simulink model "Simulink_Program"  (R2023b)
settings: Solver=FixedStepAuto  StartTime=0.0  StopTime=20  FixedStep=0.001  RelTol=1e-3
PreLoadFcn:
  | % NXP_MBDT_S32K3 callback: DO NOT REMOVE
  | mbd_s32k3.common.nxp.model_callbacks.preLoadFcn();
PostLoadFcn:
  | % NXP_MBDT_S32K3 callback: DO NOT REMOVE
  | mbd_s32k3.common.nxp.model_callbacks.postLoadFcn();
InitFcn:
  | % NXP_MBDT_S32K3 callback: DO NOT REMOVE
  | mbd_s32k3.common.nxp.model_callbacks.initFcn();
undefined symbols (not defined in the file or in its folder): battery_builder_rtmsupport, DiscIntNeverNeededParam, gcbh
libraries: nesl_utility x116, pack_105s34p_structured_with_balancing_lib x105, fl_lib x16, ee_lib x7, simulink x5, BatteryBalancing x1
(part 1 of 8)
settings Model: DataDictionary=Simulink_Program.sldd  Creator=romul  LastModifiedBy=mcmas
settings OptimizationCC: EnhancedBackFolding=on  PassReuseOutputArgsAs=Individual arguments  ZeroExternalMemoryAtStartup=off  ZeroInternalMemoryAtStartup=off  MaxStackSize=64  OptimizeBlockOrder=speed  DifferentSizesBufferReuse=on  OptimizationCustomize=off  EfficientTunableParamExpr=on
settings DebuggingCC: ParameterTunabilityLossMsg=error
settings HardwareCC: ProdBitPerPointer=32  ProdBitPerSizeT=32  ProdBitPerPtrDiffT=32  ProdLargestAtomicInteger=Long  ProdLargestAtomicFloat=Double  ProdWordSize=32  ProdHWDeviceType=ARM Compatible->ARM Cortex
settings SFSimCC: MATLABDynamicMemAlloc=off
settings RTWCC: Description=Embedded Coder  SystemTargetFile=ert.tlc  HardwareBoard=NXP S32K3xx  TemplateMakefile=ert_default_tmf  Toolchain=S32DS GCC
settings CodeAppCC: ForceParamTrailComments=on  IgnoreCustomStorageClasses=off  OperatorAnnotations=on  SimulinkDataObjDesc=on  SFDataObjDesc=on  InsertBlockDesc=on
settings ERTTargetCC: ExtModeTransport=1  ExtModeMexFile=ext_serial_win32_comm  ERTSrcFileBannerTemplate=mbd_s32k3_ert_code_template.cgt  ERTHdrFileBannerTemplate=mbd_s32k3_ert_code_template.cgt  ERTDataSrcFileTemplate=mbd_s32k3_ert_code_template.cgt  ERTDataHdrFileTemplate=mbd_s32k3_ert_code_template.cgt

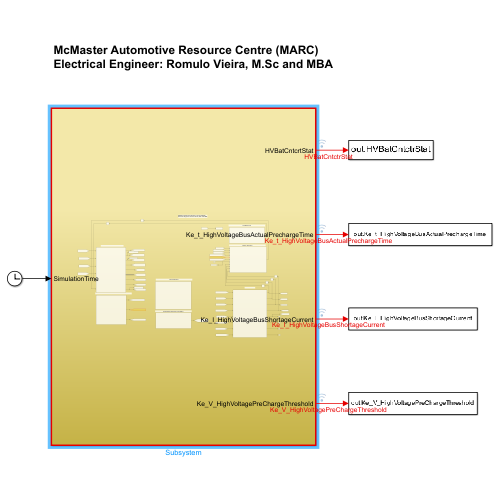
[thumbnail: Simulink's stored preview of the top-level diagram]
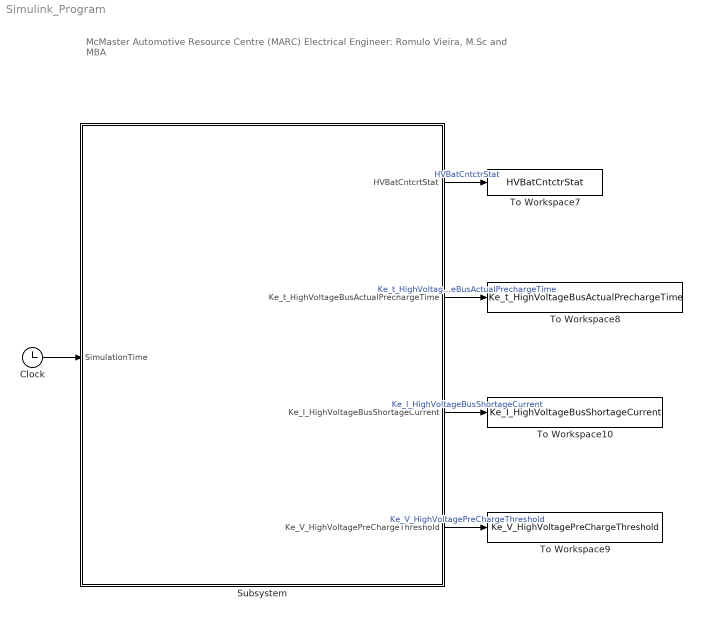
== system Simulink_Program ==
  note: McMaster Automotive Resource Centre (MARC)
      | Electrical Engineer: Romulo Vieira, M.Sc and MBA
  Clock : Clock
  Subsystem : Subsystem  TreatAsAtomicUnit=on
  To Workspace10 : ToWorkspace  VariableName=Ke_I_HighVoltageBusShortageCurrent  SampleTime=-1
  To Workspace7 : ToWorkspace  VariableName=HVBatCntctrStat  SampleTime=-1
  To Workspace8 : ToWorkspace  VariableName=Ke_t_HighVoltageBusActualPrechargeTime  SampleTime=-1
  To Workspace9 : ToWorkspace  VariableName=Ke_V_HighVoltagePreChargeThreshold  SampleTime=-1
  wires:
  Subsystem/4(Ke_V_HighVoltagePreChargeThreshold) -> To Workspace9/1  "Ke_V_HighVoltagePreChargeThreshold"
  Subsystem/2(Ke_t_HighVoltageBusActualPrechargeTime) -> To Workspace8/1  "Ke_t_HighVoltageBusActualPrechargeTime"
  Subsystem/1(HVBatCntcrtStat) -> To Workspace7/1  "HVBatCntctrStat"
  Subsystem/3(Ke_I_HighVoltageBusShortageCurrent) -> To Workspace10/1  "Ke_I_HighVoltageBusShortageCurrent"
  Clock/1 -> Subsystem/1(SimulationTime)

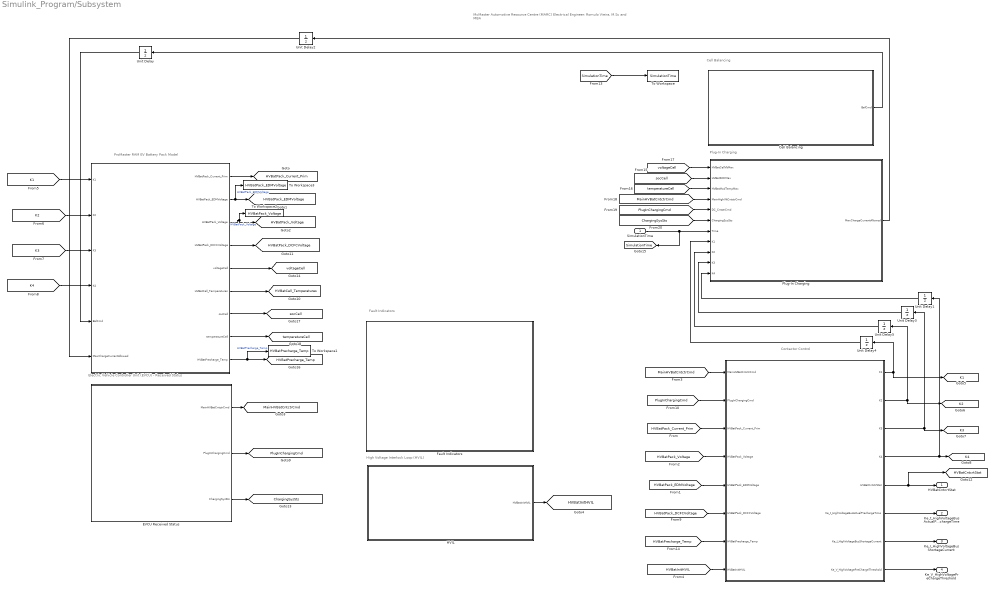
== system Simulink_Program/Subsystem ==
  note: McMaster Automotive Resource Centre (MARC)
      | Electrical Engineer: Romulo Vieira, M.Sc and MBA
  note: ProMaster RAM EV Battery Pack Model
  note: Contactor Control
  note: Plug-In Charging
  note: Cell Balancing
  note: High Voltage Interlock Loop (HVIL)
  note: Fault Indicators
  note: Electric Vehicle Controller Unit (EVCU) - Received Status
  SimulationTime : Inport
  " " : Subsystem  TreatAsAtomicUnit=on  SystemSampleTime=0.001
  "  " : Subsystem  TreatAsAtomicUnit=on  SystemSampleTime=0.001
  Cell Balancing : Subsystem
  EVCU Received Status : Subsystem
  Fault Indicators : Subsystem
  HVIL : Subsystem
  Plug-In Charging : Subsystem  TreatAsAtomicUnit=on  SystemSampleTime=0.001
  To Workspace : ToWorkspace  VariableName=SimulationTime  SampleTime=-1
  To Workspace1 : ToWorkspace  VariableName=HVBatPrecharge_Temp  SampleTime=-1
  To Workspace2 : ToWorkspace  VariableName=HVBatPack_Voltage  SampleTime=-1
  To Workspace3 : ToWorkspace  VariableName=HVBatPack_EDMVoltage  SampleTime=-1
  Unit Delay{,1..5} (6 alike) : UnitDelay  SampleTime=-1  HasFrameUpgradeWarning=on
  HVBatCntcrtStat : Outport
  Ke_t_HighVoltageBusActualPrechargeTime : Outport  Port=2
  Ke_I_HighVoltageBusShortageCurrent : Outport  Port=3
  Ke_V_HighVoltagePreChargeThreshold : Outport  Port=4
  tag scope: ChargingSysSts=global, HVBatCell_Temperatures=global, HVBatCntcrtStat=global, HVBatInitHVIL=global, HVBatPack_Current_Prim=global, HVBatPack_DCFCVoltage=global, HVBatPack_EDMVoltage=global, HVBatPack_Voltage=global, HVBatPrecharge_Temp=global, K1=global, K2=global, K3=global, K4=global, MainHVBatCntctrCmd=global, PlugInChargingCmd=global, SimulationTime=global, socCell=global, temperatureCell=global, voltageCell=global
  wires:
  "  "/5(HVBatCntctrStat) -> [HVBatCntcrtStat], HVBatCntcrtStat/1
  "  "/6(Ke_t_HighVoltageBusActualPrechargeTime) -> Ke_t_HighVoltageBusActualPrechargeTime/1
  "  "/7(Ke_I_HighVoltageBusShortageCurrent) -> Ke_I_HighVoltageBusShortageCurrent/1
  "  "/8(Ke_V_HighVoltagePreChargeThreshold) -> Ke_V_HighVoltagePreChargeThreshold/1
  " "/1(HVBatPack_Current_Prim) -> [HVBatPack_Current_Prim]
  " "/2(HVBatPack_EDMVoltage) -> To Workspace3/1, [HVBatPack_EDMVoltage]  "HVBatPack_EDMVoltage"
  " "/3(HVBatPack_Voltage) -> To Workspace2/1, [HVBatPack_Voltage]  "HVBatPack_Voltage"
  [HVBatPack_Current_Prim] -> "  "/3(HVBatPack_Current_Prim)
  [HVBatPack_EDMVoltage] -> "  "/5(HVBatPack_EDMVoltage)
  [HVBatPack_Voltage] -> "  "/4(HVBatPack_Voltage)
  EVCU Received Status/1(" MainHVBatCntctrCmd      ") -> [MainHVBatCntctrCmd]
  [MainHVBatCntctrCmd] -> "  "/1(MainHVBatCntctrCmd)
  HVIL/1("HVBatInitHVIL   ") -> [HVBatInitHVIL]
  [HVBatInitHVIL] -> "  "/8(HVBatInitHVIL)
  "  "/3(K3) -> [K3], Unit Delay3/1
  "  "/4(K4) -> Unit Delay1/1, [K4]
  [K2] -> " "/2(K2)
  [K3] -> " "/3(K3)
  [K4] -> " "/4(K4)
  " "/4(HVBatPack_DCFCVoltage) -> [HVBatPack_DCFCVoltage]
  [HVBatPack_DCFCVoltage] -> "  "/6(HVBatPack_DCFCVoltage)
  [PlugInChargingCmd] -> "  "/2(PlugInChargingCmd)
  EVCU Received Status/2(" PlugInChargingCmd      ") -> [PlugInChargingCmd]
  " "/5(voltageCell) -> [voltageCell]
  " "/6(HVBatCell_Temperatures) -> [HVBatCell_Temperatures]
  " "/9(HVBatPrecharge_Temp) -> To Workspace1/1, [HVBatPrecharge_Temp]  "HVBatPrecharge_Temp"
  [K1] -> " "/1(K1)
  [SimulationTime] -> To Workspace/1
  SimulationTime/1 -> [SimulationTime], Plug-In Charging/7(Time)
  Cell Balancing/1(BalCmd) -> Unit Delay/1
  Unit Delay/1 -> " "/5(BalCmd)
  Unit Delay1/1 -> Plug-In Charging/11(K4)
  EVCU Received Status/3(" ChargingSysSts") -> [ChargingSysSts]
  [HVBatPrecharge_Temp] -> "  "/7(HVBatPrecharge_Temp)
  Unit Delay3/1 -> Plug-In Charging/10(K3)
  Unit Delay5/1 -> Plug-In Charging/9(K2)
  "  "/2(K2) -> [K2], Unit Delay5/1
  Unit Delay4/1 -> Plug-In Charging/8(K1)
  "  "/1(K1) -> [K1], Unit Delay4/1
  " "/7(socCell) -> [socCell]
  " "/8(temperatureCell) -> [temperatureCell]
  Plug-In Charging/1(MaxChargeCurrentAllowed) -> Unit Delay2/1
  Unit Delay2/1 -> " "/6(MaxChargeCurrentAllowed)
  [voltageCell] -> Plug-In Charging/1(HVBatCellVltMax)
  [socCell] -> Plug-In Charging/2(HVBatSOCMax)
  [temperatureCell] -> Plug-In Charging/3(HVBatModTempMax)
  [MainHVBatCntctrCmd] -> Plug-In Charging/4(MainHighVltCntctrCmd)
  [PlugInChargingCmd] -> Plug-In Charging/5(DC_CntctrCmd)
  [ChargingSysSts] -> Plug-In Charging/6(ChargingSysSts)

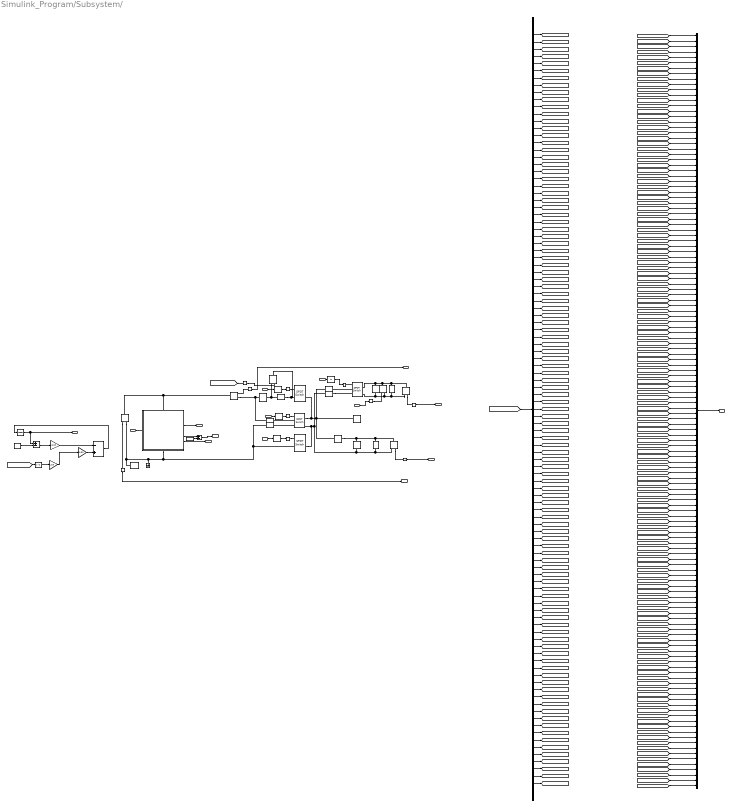
== system Simulink_Program/Subsystem/  ==
  note: McMaster Automotive Resource Centre (MARC)
      | Electrical Engineer: Romulo Vieira, M.Sc and MBA
  note: Bus Creator for cell temperatures
  note: Demux to separate 105 temperatures
  note: Measuring precharge resistor temperature
      | 1. Measure Voltage Across the Precharge Resistor using a voltage sensor.
      | 2. Calculate Power Dissipated in the Resistor. Use P(t) = V(t)^2 / R where precharge resistance, R = 25 ohm
      | 3. Model the Thermal Behaviour. Use dT/dt = P(t)/C - (T(t) - T_ambient)/(R_th*C) where thermal capacitance, C = 50 J/deg C, thermal resistance, R_th = 2 deg C/W and ambient temperature, T_ambient = 25 deg C
      | 4. Use an integrator to output the final temperature value.
  note: Input
      | precharge_resistor_voltage
      |
      | Output
      | precharge_resistor_temperature
  K1 : Inport
  K2 : Inport  Port=2
  K3 : Inport  Port=3
  K4 : Inport  Port=4
  BalCmd : Inport  Port=5
  MaxChargeCurrentAllowed : Inport  Port=6
  "   " : nesl_utility/PS-Simulink Converter  PhysicalDomain=network_engine_domain  SubClassName=ps_output  LeftPortType=input  RightPortType=output  PseudoPeriodic=off  Frequencies=[]  VectorFormat=inherit  Unit=inherit  AffineConversion=off
  " " : nesl_utility/PS-Simulink Converter  PhysicalDomain=network_engine_domain  SubClassName=ps_output  LeftPortType=input  RightPortType=output  PseudoPeriodic=off  Frequencies=[]  VectorFormat=inherit  Unit=inherit  AffineConversion=off
  " 1" : nesl_utility/PS-Simulink Converter  PhysicalDomain=network_engine_domain  SubClassName=ps_output  LeftPortType=input  RightPortType=output  PseudoPeriodic=off  Frequencies=[]  VectorFormat=inherit  Unit=inherit  AffineConversion=off
  1/(R_th*C) : Gain  Gain=1/100
  1/C : Gain  Gain=1/50
  1/R : Gain  Gain=1/25
  BusCreator_Temp : BusCreator  Inputs=140
  Capacitor : fl_lib/Electrical/Electrical Elements/Capacitor  c=750e-6 F  r=1e-6 Ohm  g=0 1/Ohm  i_specify=off  i_priority=None  i=0 A  i_nominal_specify=off  i_nominal_unit=A  i_nominal_value=1  v_specify=off  v_priority=None  v=0 V  v_nominal_specify=off  v_nominal_unit=V  v_nominal_value=1  vc_specify=off  vc_priority=High  vc=0 V  vc_nominal_specify=off  vc_nominal_unit=V  vc_nominal_value=1
  Capacitor Bank : fl_lib/Electrical/Electrical Elements/Capacitor  c=750e-6 F  r=1e-6 Ohm  g=0 1/Ohm  i_specify=off  i_priority=None  i=0 A  i_nominal_specify=off  i_nominal_unit=A  i_nominal_value=1  v_specify=off  v_priority=None  v=0 V  v_nominal_specify=off  v_nominal_unit=V  v_nominal_value=1  vc_specify=off  vc_priority=High  vc=441 V  vc_nominal_specify=off  vc_nominal_unit=V  vc_nominal_value=1
  Constant : Constant  Value=273.15
  Controlled Current Source : fl_lib/Electrical/Electrical Sources/Controlled Current Source
  Current Sensor : fl_lib/Electrical/Electrical Sensors/Current Sensor
  Delay : Delay  DelayLength=1
  Delay4 : Delay  DelayLength=1
  Delay5 : Delay  DelayLength=1
  Demux1 : Demux  Outputs=105
  Discrete-Time Integrator : DiscreteIntegrator  InitialCondition=25  SampleTime=0.001  ICPrevOutput=DiscIntNeverNeededParam  ICPrevScaledInput=DiscIntNeverNeededParam
  F2 - PDC Fuse : ee_lib/Switches & Breakers/Fuse  prm=1  I_rated=125 A  melt_margin=1  T_fuse=0 s  I2t=1 A^2*s  I_vec=[1.9, 2, 2.5, 30] A  t_vec=[10000, 1, .3, .001] s  R_closed=0.01 Ohm  G_open=1e-8 1/Ohm  exposemeasurement=ee.enum.exposemeasurement.hidden
      settings: InternalSimscapePortConfiguration="{\"Bottom\":[],\"Left\":[{\"id\":\"p\",\"label\":\"\",\"type\":\"foundation.electrical.electrical\"}],\"Right\":[{\"id\":\"n\",\"label\":\"\",\"type\":\"foundation.electrical.electrical\"}],\"Top\":[]}"
  F3 - EDM Fuse : ee_lib/Switches & Breakers/Fuse  prm=1  I_rated=600 A  melt_margin=1  T_fuse=0 s  I2t=1 A^2*s  I_vec=[1.9, 2, 2.5, 30] A  t_vec=[10000, 1, .3, .001] s  R_closed=0.01 Ohm  G_open=1e-8 1/Ohm  exposemeasurement=ee.enum.exposemeasurement.hidden
      settings: InternalSimscapePortConfiguration="{\"Bottom\":[],\"Left\":[{\"id\":\"p\",\"label\":\"\",\"type\":\"foundation.electrical.electrical\"}],\"Right\":[{\"id\":\"n\",\"label\":\"\",\"type\":\"foundation.electrical.electrical\"}],\"Top\":[]}"
  Ground : fl_lib/Electrical/Electrical Elements/Electrical Reference
  K1 - Main Contactor : ee_lib/Switches & Breakers/DPDT Switch  R_closed=0.01 Ohm  G_open=1e-6 S  Threshold=0.5 V  Width=0 V  model_dynamics=0  t_on=1e-3 s  t_off=1e-3 s  initial_vT=0 V
      settings: InternalSimscapePortConfiguration="{\"Bottom\":[],\"Left\":[{\"id\":\"vT\",\"label\":\"vT\",\"type\":\"input\"},{\"id\":\"p1\",\"label\":\"\",\"type\":\"foundation.electrical.electrical\"},{\"id\":\"p2\",\"label\":\"\",\"type\":\"foundation.electrical.electrical\"}],\"Right\":[{\"id\":\"n11\",\"label\":\"\",\"type\":\"foundation.electrical.electrical\"},{\"id\":\"n12\",\"label\":\"\",\"type\":\"foundation.electrical.electrical\"},{\"id\":\"n21\",\"label\":\"\",\"type\":\"foundation.electrical.electrical\"},{\"id\":\"n22\",\"label\":\"\",\"type\":\"foundation.electrical.electrical\"}],\"Top\":[]}"
  K2 - Precharge Relay (-) : ee_lib/Switches & Breakers/SPDT Switch  R_closed=0.01 Ohm  G_open=1e-6 S  Threshold=0.5 V  Width=0 V  model_dynamics=0  t_on=1e-3 s  t_off=1e-3 s  initial_vT=0 V
  K3 - Precharge Relay (+) : ee_lib/Switches & Breakers/SPDT Switch  R_closed=0.01 Ohm  G_open=1e-6 S  Threshold=0.5 V  Width=0 V  model_dynamics=0  t_on=1e-3 s  t_off=1e-3 s  initial_vT=0 V
  K4 - DCFC Contactor : ee_lib/Switches & Breakers/DPDT Switch  R_closed=0.01 Ohm  G_open=1e-6 S  Threshold=0.5 V  Width=0 V  model_dynamics=0  t_on=1e-3 s  t_off=1e-3 s  initial_vT=0 V
      settings: InternalSimscapePortConfiguration="{\"Bottom\":[],\"Left\":[{\"id\":\"vT\",\"label\":\"vT\",\"type\":\"input\"},{\"id\":\"p1\",\"label\":\"\",\"type\":\"foundation.electrical.electrical\"},{\"id\":\"p2\",\"label\":\"\",\"type\":\"foundation.electrical.electrical\"}],\"Right\":[{\"id\":\"n11\",\"label\":\"\",\"type\":\"foundation.electrical.electrical\"},{\"id\":\"n12\",\"label\":\"\",\"type\":\"foundation.electrical.electrical\"},{\"id\":\"n21\",\"label\":\"\",\"type\":\"foundation.electrical.electrical\"},{\"id\":\"n22\",\"label\":\"\",\"type\":\"foundation.electrical.electrical\"}],\"Top\":[]}"
  K4 Resistor (Pole 1) : fl_lib/Electrical/Electrical Elements/Resistor  R=0.0003 Ohm  i_specify=off  i_priority=None  i=0 A  i_nominal_specify=off  i_nominal_unit=A  i_nominal_value=1  v_specify=off  v_priority=None  v=0 V  v_nominal_specify=off  v_nominal_unit=V  v_nominal_value=1
  K4 Resistor (Pole 2) : fl_lib/Electrical/Electrical Elements/Resistor  R=0.0003 Ohm  i_specify=off  i_priority=None  i=0 A  i_nominal_specify=off  i_nominal_unit=A  i_nominal_value=1  v_specify=off  v_priority=None  v=0 V  v_nominal_specify=off  v_nominal_unit=V  v_nominal_value=1
  PS-Simulink 1 : nesl_utility/PS-Simulink Converter  PhysicalDomain=network_engine_domain  SubClassName=ps_output  LeftPortType=input  RightPortType=output  PseudoPeriodic=off  Frequencies=[]  VectorFormat=inherit  Unit=inherit  AffineConversion=off
  PS-Simulink Converter : nesl_utility/PS-Simulink Converter  PhysicalDomain=network_engine_domain  SubClassName=ps_output  LeftPortType=input  RightPortType=output  PseudoPeriodic=off  Frequencies=[]  VectorFormat=inherit  Unit=inherit  AffineConversion=off
  Pack1 : Subsystem  LoadFcn=battery_builder_rtmsupport('loadfcn',gcbh);
      settings: CopyFcn=battery_builder_rtmsupport('copyfcn',gcbh);  PreCopyFcn=battery_builder_rtmsupport('precopyfcn',gcbh);  PreDeleteFcn=battery_builder_rtmsupport('predeletefcn',gcbh);  PortSchema="{\"entries\":[{\"content\":{\"sides\":[{\"content\":{\"connectorIds\":[\"LConn1\"]},\"type\":\"ConnectorPlacement.EquallySpacedRectSide\",\"uuid\":\"5e007e92-2246-4b9f-a838-21fe4b771105\"},{\"content\":{\"connectorIds\":[\"RConn1\"],\"side\":\"RIGHT\"},\"type\":\"ConnectorPlacement.EquallySpacedRectSide\",\"uuid\":\"c53269da-b12c-4944-8bcf-f548990142fd\"},{\"content\":{\"connectorIds\":[\"In1\"],\"side\":\"TOP\"},\"type\":\"ConnectorPlacement.EquallySpacedRectSide\",\"uuid\":\"229c8070-2bae-4d06-bc1c-b3e24a21fda1\"},{\"content\":{\"connectorIds\":[\"Out1\",\"Out2\",\"Out3\",\"Out4\",\"Out5\",\"Out6\",\"Out7\"],\"side\":\"BOTTOM\"},\"type\":\"ConnectorPlacement.EquallySpacedRectSide\",\"uuid\":\"01f05c69-bd27-4a3f-8a69-5e0eef156d08\"}]},\"type\":\"ConnectorPlacement.EquallySpacedConnector\",\"uuid\":\"d4cf6711-9d91-4f90-9da8-75b40740f7c2\"}],\"packageUris\":[\"http://schema.mathworks.com/mf0/Connector/R2020b\"],\"version\":\"1.0\"}"
      mask Display: image('$imagefile')
  Precharge Fuse : ee_lib/Switches & Breakers/Fuse  prm=1  I_rated=600 A  melt_margin=1  T_fuse=0 s  I2t=1 A^2*s  I_vec=[1.9, 2, 2.5, 30] A  t_vec=[10000, 1, .3, .001] s  R_closed=0.01 Ohm  G_open=1e-8 1/Ohm  exposemeasurement=ee.enum.exposemeasurement.hidden
      settings: InternalSimscapePortConfiguration="{\"Bottom\":[],\"Left\":[{\"id\":\"p\",\"label\":\"\",\"type\":\"foundation.electrical.electrical\"}],\"Right\":[{\"id\":\"n\",\"label\":\"\",\"type\":\"foundation.electrical.electrical\"}],\"Top\":[]}"
  Precharge Resistor : fl_lib/Electrical/Electrical Elements/Resistor  R=25 Ohm  i_specify=off  i_priority=None  i=0 A  i_nominal_specify=off  i_nominal_unit=A  i_nominal_value=1  v_specify=off  v_priority=None  v=0 V  v_nominal_specify=off  v_nominal_unit=V  v_nominal_value=1
  Resistor : fl_lib/Electrical/Electrical Elements/Resistor  R=1 Ohm  i_specify=off  i_priority=None  i=0 A  i_nominal_specify=off  i_nominal_unit=A  i_nominal_value=1  v_specify=off  v_priority=None  v=0 V  v_nominal_specify=off  v_nominal_unit=V  v_nominal_value=1
  Resistor1 : fl_lib/Electrical/Electrical Elements/Resistor  R=1 Ohm  i_specify=off  i_priority=None  i=0 A  i_nominal_specify=off  i_nominal_unit=A  i_nominal_value=1  v_specify=off  v_priority=None  v=0 V  v_nominal_specify=off  v_nominal_unit=V  v_nominal_value=1
  Resistor2 : fl_lib/Electrical/Electrical Elements/Resistor  R=40 kOhm  i_specify=off  i_priority=None  i=0 A  i_nominal_specify=off  i_nominal_unit=A  i_nominal_value=1  v_specify=off  v_priority=None  v=0 V  v_nominal_specify=off  v_nominal_unit=V  v_nominal_value=1
  Resistor3 : fl_lib/Electrical/Electrical Elements/Resistor  R=40 kOhm  i_specify=off  i_priority=None  i=0 A  i_nominal_specify=off  i_nominal_unit=A  i_nominal_value=1  v_specify=off  v_priority=None  v=0 V  v_nominal_specify=off  v_nominal_unit=V  v_nominal_value=1
  Simulink-PS Converter{,1..4} (5 alike) : nesl_utility/Simulink-PS Converter  PhysicalDomain=network_engine_domain  SubClassName=ps_input  LeftPortType=input  RightPortType=output  PseudoPeriodic=off  Frequencies=[]  NoiseDistribution=none  NoiseParameters=[]  Unit=1  AffineConversion=off  FilteringAndDerivatives=provide  SimscapeFilterOrder=1  InputFilterTimeConstant=0.001  UdotUserProvided=0
  Solver Configuration : nesl_utility/Solver Configuration  PhysicalDomain=network_engine_domain  SubClassName=solver  LeftPortType=input  RightPortType=generic  Tones=[]  Harmonics=[]  SimulateNoise=off  Profile=off  UseLocalSampling=off  EnableSwitchedLinearOptims=on  FrequencyDomain=off  UseCCode=off  RelTol=0.001  AbsTol=0.001  MinStep=1e-09  ToWorkspace=off  RevertToSquareIcSolve=off  NumHomotopyIterations=100  PhasorMode=off  PhasorModeNumHarmonics=1  ConsistencySolver=NEWTON_XTOL_AFTER_FTOL  IndexReductionMethod=DerivativeReplacement  DoDC=off  ResidualTolerance=1e-09  ConsistencyTolSource=GLOBAL  ConsistencyAbsTol=0.001  ConsistencyRelTol=0.001  ConsistencyTolFactor=0.001  UseLocalSolver=on  LocalSolverChoice=NE_BACKWARD_EULER_ADVANCER  ResolveIndetEquations=on  FunctionEvalNumThread=1  LocalSolverSampleTime=0.001  DoFixedCost=on  MaxNonlinIter=3  MaxModeIter=2  ComputeImpulses=off  ImpulseIterations=2  LinearAlgebra=auto  LinearAlgebraNumThread=0  EquationFormulation=NE_TIME_EF  DelaysMemoryBudget=1024  AutomaticFiltering=off  FilteringTimeConstant=0.001  PartitionStorageMethod=AS_NEEDED  PartitionMemoryBudget=1024  PartitionMethod=ROBUST  MultibodyUseLocalSolver=off  MultibodyLocalSolverChoice=ODE1  MultibodyLocalSolverSampleTime=0.001
  Subtract : Sum  Inputs=-+
  Subtract1 : Sum  Inputs=+-
  T(t) - T_ambient : Sum  Inputs=+-
  T_ambient : Constant  Value=25
  Unit Delay : UnitDelay  SampleTime=-1  HasFrameUpgradeWarning=on
  V^2 : Math  Operator=square
  Voltage Sensor{,1..3} (4 alike) : fl_lib/Electrical/Electrical Sensors/Voltage Sensor
  HVBatPack_Current_Prim : Outport
  HVBatPack_EDMVoltage : Outport  Port=2
  HVBatPack_Voltage : Outport  Port=3
  HVBatPack_DCFCVoltage : Outport  Port=4
  voltageCell : Outport  Port=5
  HVBatCell_Temperatures : Outport  Port=6
  socCell : Outport  Port=7
  temperatureCell : Outport  Port=8
  HVBatPrecharge_Temp : Outport  Port=9
  tag scope: HVBatCell_Temperature001=global, HVBatCell_Temperature002=global, HVBatCell_Temperature003=global, HVBatCell_Temperature004=global, HVBatCell_Temperature005=global, HVBatCell_Temperature006=global, HVBatCell_Temperature007=global, HVBatCell_Temperature008=global, HVBatCell_Temperature009=global, HVBatCell_Temperature010=global, HVBatCell_Temperature011=global, HVBatCell_Temperature012=global, HVBatCell_Temperature013=global, HVBatCell_Temperature014=global, HVBatCell_Temperature015=global, HVBatCell_Temperature016=global, HVBatCell_Temperature017=global, HVBatCell_Temperature018=global, HVBatCell_Temperature019=global, HVBatCell_Temperature020=global, HVBatCell_Temperature021=global, HVBatCell_Temperature022=global, HVBatCell_Temperature023=global, HVBatCell_Temperature024=global, HVBatCell_Temperature025=global, HVBatCell_Temperature026=global, HVBatCell_Temperature027=global, HVBatCell_Temperature028=global, HVBatCell_Temperature029=global, HVBatCell_Temperature030=global, HVBatCell_Temperature031=global, HVBatCell_Temperature032=global, HVBatCell_Temperature033=global, HVBatCell_Temperature034=global, HVBatCell_Temperature035=global, HVBatCell_Temperature036=global, HVBatCell_Temperature037=global, HVBatCell_Temperature038=global, HVBatCell_Temperature039=global, HVBatCell_Temperature040=global, HVBatCell_Temperature041=global, HVBatCell_Temperature042=global, HVBatCell_Temperature043=global, HVBatCell_Temperature044=global, HVBatCell_Temperature045=global, HVBatCell_Temperature046=global, HVBatCell_Temperature047=global, HVBatCell_Temperature048=global, HVBatCell_Temperature049=global, HVBatCell_Temperature050=global, HVBatCell_Temperature051=global, HVBatCell_Temperature052=global, HVBatCell_Temperature053=global, HVBatCell_Temperature054=global, HVBatCell_Temperature055=global, HVBatCell_Temperature056=global, HVBatCell_Temperature057=global, HVBatCell_Temperature058=global, HVBatCell_Temperature059=global, HVBatCell_Temperature060=global, HVBatCell_Temperature061=global, HVBatCell_Temperature062=global, HVBatCell_Temperature063=global, HVBatCell_Temperature064=global, HVBatCell_Temperature065=global, HVBatCell_Temperature066=global, HVBatCell_Temperature067=global, HVBatCell_Temperature068=global, HVBatCell_Temperature069=global, HVBatCell_Temperature070=global, HVBatCell_Temperature071=global, HVBatCell_Temperature072=global, HVBatCell_Temperature073=global, HVBatCell_Temperature074=global, HVBatCell_Temperature075=global, HVBatCell_Temperature076=global, HVBatCell_Temperature077=global, HVBatCell_Temperature078=global, HVBatCell_Temperature079=global, HVBatCell_Temperature080=global, HVBatCell_Temperature081=global, HVBatCell_Temperature082=global, HVBatCell_Temperature083=global, HVBatCell_Temperature084=global, HVBatCell_Temperature085=global, HVBatCell_Temperature086=global, HVBatCell_Temperature087=global, HVBatCell_Temperature088=global, HVBatCell_Temperature089=global, HVBatCell_Temperature090=global, HVBatCell_Temperature091=global, HVBatCell_Temperature092=global, HVBatCell_Temperature093=global, HVBatCell_Temperature094=global, HVBatCell_Temperature095=global, HVBatCell_Temperature096=global, HVBatCell_Temperature097=global, HVBatCell_Temperature098=global, HVBatCell_Temperature099=global, HVBatCell_Temperature100=global, HVBatCell_Temperature101=global, HVBatCell_Temperature102=global, HVBatCell_Temperature103=global, HVBatCell_Temperature104=global, HVBatCell_Temperature105=global, HVBatPrecharge_Voltage=global
  wires:
  "   "/1 -> HVBatPack_Current_Prim/1
  K2/1 -> Delay5/1
  K3/1 -> Delay4/1
  Delay4/1 -> Simulink-PS Converter/1
  Delay5/1 -> Simulink-PS Converter2/1
  PS-Simulink 1/1 -> HVBatPack_Voltage/1
  Pack1/6(vCell) -> voltageCell/1
  PS-Simulink Converter/1 -> [HVBatPrecharge_Voltage]
  Pack1/5(temperatureCell) -> Subtract1/1
  Constant/1 -> Subtract1/2
  [HVBatCell_Temperature018] -> BusCreator_Temp/18  "Cell Temperature 18"
  [HVBatCell_Temperature031] -> BusCreator_Temp/31  "Cell Temperature 31"
  [HVBatCell_Temperature042] -> BusCreator_Temp/42  "Cell Temperature 42"
  [HVBatCell_Temperature094] -> BusCreator_Temp/94  "Cell Temperature 94"
  Demux1/20 -> [HVBatCell_Temperature020]
  [HVBatCell_Temperature082] -> BusCreator_Temp/82  "Cell Temperature 82"
  [HVBatCell_Temperature035] -> BusCreator_Temp/35  "Cell Temperature 35"
  [HVBatCell_Temperature064] -> BusCreator_Temp/64  "Cell Temperature 64"
  Demux1/79 -> [HVBatCell_Temperature079]
  Demux1/68 -> [HVBatCell_Temperature068]
  Demux1/8 -> [HVBatCell_Temperature008]
  [HVBatCell_Temperature036] -> BusCreator_Temp/36  "Cell Temperature 36"
  [HVBatCell_Temperature039] -> BusCreator_Temp/39  "Cell Temperature 39"
  Demux1/29 -> [HVBatCell_Temperature029]
  [HVBatCell_Temperature041] -> BusCreator_Temp/41  "Cell Temperature 41"
  Demux1/67 -> [HVBatCell_Temperature067]
  Demux1/13 -> [HVBatCell_Temperature013]
  [HVBatCell_Temperature092] -> BusCreator_Temp/92  "Cell Temperature 92"
  [HVBatCell_Temperature087] -> BusCreator_Temp/87  "Cell Temperature 87"
  Demux1/84 -> [HVBatCell_Temperature084]
  Demux1/75 -> [HVBatCell_Temperature075]
  [HVBatCell_Temperature071] -> BusCreator_Temp/71  "Cell Temperature 71"
  Demux1/9 -> [HVBatCell_Temperature009]
  Demux1/14 -> [HVBatCell_Temperature014]
  Demux1/11 -> [HVBatCell_Temperature011]
  [HVBatCell_Temperature016] -> BusCreator_Temp/16  "Cell Temperature 16"
  [HVBatCell_Temperature011] -> BusCreator_Temp/11  "Cell Temperature 11"
  Demux1/38 -> [HVBatCell_Temperature038]
  Demux1/41 -> [HVBatCell_Temperature041]
  [HVBatCell_Temperature086] -> BusCreator_Temp/86  "Cell Temperature 86"
  [HVBatCell_Temperature070] -> BusCreator_Temp/70  "Cell Temperature 70"
  [HVBatCell_Temperature057] -> BusCreator_Temp/57  "Cell Temperature 57"
  Demux1/40 -> [HVBatCell_Temperature040]
  Demux1/101 -> [HVBatCell_Temperature101]
  Demux1/100 -> [HVBatCell_Temperature100]
  Demux1/55 -> [HVBatCell_Temperature055]
  Demux1/77 -> [HVBatCell_Temperature077]
  Demux1/23 -> [HVBatCell_Temperature023]
  Demux1/5 -> [HVBatCell_Temperature005]
  Demux1/48 -> [HVBatCell_Temperature048]
  Demux1/76 -> [HVBatCell_Temperature076]
  Demux1/7 -> [HVBatCell_Temperature007]
  Demux1/95 -> [HVBatCell_Temperature095]
  Demux1/34 -> [HVBatCell_Temperature034]
  [HVBatCell_Temperature044] -> BusCreator_Temp/44  "Cell Temperature 44"
  [HVBatCell_Temperature097] -> BusCreator_Temp/97  "Cell Temperature 97"
  [HVBatCell_Temperature026] -> BusCreator_Temp/26  "Cell Temperature 26"
  Demux1/62 -> [HVBatCell_Temperature062]
  [HVBatCell_Temperature004] -> BusCreator_Temp/4  "Cell Temperature 4"
  [HVBatCell_Temperature007] -> BusCreator_Temp/7  "Cell Temperature 7"
  [HVBatCell_Temperature048] -> BusCreator_Temp/48  "Cell Temperature 48"
  Demux1/88 -> [HVBatCell_Temperature088]
  [HVBatCell_Temperature034] -> BusCreator_Temp/34  "Cell Temperature 34"
  [HVBatCell_Temperature028] -> BusCreator_Temp/28  "Cell Temperature 28"
  Demux1/35 -> [HVBatCell_Temperature035]
  Demux1/30 -> [HVBatCell_Temperature030]
  Demux1/86 -> [HVBatCell_Temperature086]
  Demux1/51 -> [HVBatCell_Temperature051]
  Demux1/39 -> [HVBatCell_Temperature039]
  Demux1/99 -> [HVBatCell_Temperature099]
  Demux1/85 -> [HVBatCell_Temperature085]
  [HVBatCell_Temperatures_pack] -> Demux1/1
  [HVBatCell_Temperature005] -> BusCreator_Temp/5  "Cell Temperature 5"
  [HVBatCell_Temperature095] -> BusCreator_Temp/95  "Cell Temperature 95"
  [HVBatCell_Temperature003] -> BusCreator_Temp/3  "Cell Temperature 3"
  Demux1/28 -> [HVBatCell_Temperature028]
  [HVBatCell_Temperature046] -> BusCreator_Temp/46  "Cell Temperature 46"
  [HVBatCell_Temperature051] -> BusCreator_Temp/51  "Cell Temperature 51"
  [HVBatCell_Temperature098] -> BusCreator_Temp/98  "Cell Temperature 98"
  Demux1/102 -> [HVBatCell_Temperature102]
  [HVBatCell_Temperature105] -> BusCreator_Temp/105  "Cell Temperature 105"
  Demux1/80 -> [HVBatCell_Temperature080]
  Demux1/21 -> [HVBatCell_Temperature021]
  [HVBatCell_Temperature032] -> BusCreator_Temp/32  "Cell Temperature 32"
  [HVBatCell_Temperature100] -> BusCreator_Temp/100  "Cell Temperature 100"
  [HVBatCell_Temperature076] -> BusCreator_Temp/76  "Cell Temperature 76"
  Demux1/74 -> [HVBatCell_Temperature074]
  Demux1/97 -> [HVBatCell_Temperature097]
  Demux1/47 -> [HVBatCell_Temperature047]
  Demux1/36 -> [HVBatCell_Temperature036]
  [HVBatCell_Temperature058] -> BusCreator_Temp/58  "Cell Temperature 58"
  [HVBatCell_Temperature012] -> BusCreator_Temp/12  "Cell Temperature 12"
  [HVBatCell_Temperature008] -> BusCreator_Temp/8  "Cell Temperature 8"
  [HVBatCell_Temperature099] -> BusCreator_Temp/99  "Cell Temperature 99"
  Demux1/31 -> [HVBatCell_Temperature031]
  [HVBatCell_Temperature055] -> BusCreator_Temp/55  "Cell Temperature 55"
  [HVBatCell_Temperature088] -> BusCreator_Temp/88  "Cell Temperature 88"
  Demux1/46 -> [HVBatCell_Temperature046]
  [HVBatCell_Temperature103] -> BusCreator_Temp/103  "Cell Temperature 103"
  [HVBatCell_Temperature083] -> BusCreator_Temp/83  "Cell Temperature 83"
  Demux1/91 -> [HVBatCell_Temperature091]
  [HVBatCell_Temperature052] -> BusCreator_Temp/52  "Cell Temperature 52"
  Demux1/57 -> [HVBatCell_Temperature057]
  [HVBatCell_Temperature054] -> BusCreator_Temp/54  "Cell Temperature 54"
  Demux1/37 -> [HVBatCell_Temperature037]
  Demux1/50 -> [HVBatCell_Temperature050]
  Demux1/104 -> [HVBatCell_Temperature104]
  Demux1/42 -> [HVBatCell_Temperature042]
  Demux1/90 -> [HVBatCell_Temperature090]
  Demux1/73 -> [HVBatCell_Temperature073]
  [HVBatCell_Temperature073] -> BusCreator_Temp/73  "Cell Temperature 73"
  [HVBatCell_Temperature043] -> BusCreator_Temp/43  "Cell Temperature 43"
  Demux1/26 -> [HVBatCell_Temperature026]
  [HVBatCell_Temperature049] -> BusCreator_Temp/49  "Cell Temperature 49"
  [HVBatCell_Temperature066] -> BusCreator_Temp/66  "Cell Temperature 66"
  [HVBatCell_Temperature075] -> BusCreator_Temp/75  "Cell Temperature 75"
  [HVBatCell_Temperature096] -> BusCreator_Temp/96  "Cell Temperature 96"
  BusCreator_Temp/1 -> HVBatCell_Temperatures/1
  [HVBatCell_Temperature025] -> BusCreator_Temp/25  "Cell Temperature 25"
  Demux1/18 -> [HVBatCell_Temperature018]
  [HVBatCell_Temperature009] -> BusCreator_Temp/9  "Cell Temperature 9"
  [HVBatCell_Temperature047] -> BusCreator_Temp/47  "Cell Temperature 47"
  Demux1/64 -> [HVBatCell_Temperature064]
  [HVBatCell_Temperature084] -> BusCreator_Temp/84  "Cell Temperature 84"
  Demux1/83 -> [HVBatCell_Temperature083]
  [HVBatCell_Temperature001] -> BusCreator_Temp/1  "Cell Temperature 1"
  Demux1/56 -> [HVBatCell_Temperature056]
  Demux1/25 -> [HVBatCell_Temperature025]
  [HVBatCell_Temperature027] -> BusCreator_Temp/27  "Cell Temperature 27"
  [HVBatCell_Temperature104] -> BusCreator_Temp/104  "Cell Temperature 104"
  [HVBatCell_Temperature017] -> BusCreator_Temp/17  "Cell Temperature 17"
  Demux1/103 -> [HVBatCell_Temperature103]
  Demux1/44 -> [HVBatCell_Temperature044]
  [HVBatCell_Temperature062] -> BusCreator_Temp/62  "Cell Temperature 62"
  [HVBatCell_Temperature033] -> BusCreator_Temp/33  "Cell Temperature 33"
  [HVBatCell_Temperature022] -> BusCreator_Temp/22  "Cell Temperature 22"
  [HVBatCell_Temperature021] -> BusCreator_Temp/21  "Cell Temperature 21"
  Demux1/59 -> [HVBatCell_Temperature059]
  [HVBatCell_Temperature056] -> BusCreator_Temp/56  "Cell Temperature 56"
  Demux1/71 -> [HVBatCell_Temperature071]
  [HVBatCell_Temperature093] -> BusCreator_Temp/93  "Cell Temperature 93"
  Demux1/24 -> [HVBatCell_Temperature024]
  Demux1/69 -> [HVBatCell_Temperature069]
  [HVBatCell_Temperature045] -> BusCreator_Temp/45  "Cell Temperature 45"
  Demux1/60 -> [HVBatCell_Temperature060]
  Demux1/87 -> [HVBatCell_Temperature087]
  Demux1/6 -> [HVBatCell_Temperature006]
  [HVBatCell_Temperature006] -> BusCreator_Temp/6  "Cell Temperature 6"
  Demux1/1 -> [HVBatCell_Temperature001]
  [HVBatCell_Temperature020] -> BusCreator_Temp/20  "Cell Temperature 20"
  Demux1/22 -> [HVBatCell_Temperature022]
  Demux1/32 -> [HVBatCell_Temperature032]
  Demux1/54 -> [HVBatCell_Temperature054]
  Demux1/49 -> [HVBatCell_Temperature049]
  Demux1/81 -> [HVBatCell_Temperature081]
  [HVBatCell_Temperature063] -> BusCreator_Temp/63  "Cell Temperature 63"
  [HVBatCell_Temperature102] -> BusCreator_Temp/102  "Cell Temperature 102"
  Demux1/98 -> [HVBatCell_Temperature098]
  Demux1/89 -> [HVBatCell_Temperature089]
  [HVBatCell_Temperature015] -> BusCreator_Temp/15  "Cell Temperature 15"
  Demux1/61 -> [HVBatCell_Temperature061]
  Demux1/16 -> [HVBatCell_Temperature016]
  Demux1/63 -> [HVBatCell_Temperature063]
  Demux1/53 -> [HVBatCell_Temperature053]
  Demux1/10 -> [HVBatCell_Temperature010]
  Demux1/66 -> [HVBatCell_Temperature066]
  [HVBatCell_Temperature065] -> BusCreator_Temp/65  "Cell Temperature 65"
  [HVBatCell_Temperature077] -> BusCreator_Temp/77  "Cell Temperature 77"
  Demux1/72 -> [HVBatCell_Temperature072]
  Demux1/4 -> [HVBatCell_Temperature004]
  Demux1/45 -> [HVBatCell_Temperature045]
  [HVBatCell_Temperature013] -> BusCreator_Temp/13  "Cell Temperature 13"
  [HVBatCell_Temperature101] -> BusCreator_Temp/101  "Cell Temperature 101"
  [HVBatCell_Temperature085] -> BusCreator_Temp/85  "Cell Temperature 85"
  [HVBatCell_Temperature061] -> BusCreator_Temp/61  "Cell Temperature 61"
  [HVBatCell_Temperature038] -> BusCreator_Temp/38  "Cell Temperature 38"
  Demux1/12 -> [HVBatCell_Temperature012]
  [HVBatCell_Temperature072] -> BusCreator_Temp/72  "Cell Temperature 72"
  [HVBatCell_Temperature081] -> BusCreator_Temp/81  "Cell Temperature 81"
  Demux1/33 -> [HVBatCell_Temperature033]
  [HVBatCell_Temperature078] -> BusCreator_Temp/78  "Cell Temperature 78"
  [HVBatCell_Temperature040] -> BusCreator_Temp/40  "Cell Temperature 40"
  [HVBatCell_Temperature090] -> BusCreator_Temp/90  "Cell Temperature 90"
  Demux1/2 -> [HVBatCell_Temperature002]
  [HVBatCell_Temperature037] -> BusCreator_Temp/37  "Cell Temperature 37"
  Demux1/70 -> [HVBatCell_Temperature070]
  [HVBatCell_Temperature010] -> BusCreator_Temp/10  "Cell Temperature 10"
  Demux1/17 -> [HVBatCell_Temperature017]
  [HVBatCell_Temperature074] -> BusCreator_Temp/74  "Cell Temperature 74"
  Demux1/92 -> [HVBatCell_Temperature092]
  [HVBatCell_Temperature024] -> BusCreator_Temp/24  "Cell Temperature 24"
  [HVBatCell_Temperature050] -> BusCreator_Temp/50  "Cell Temperature 50"
  [HVBatCell_Temperature023] -> BusCreator_Temp/23  "Cell Temperature 23"
  [HVBatCell_Temperature029] -> BusCreator_Temp/29  "Cell Temperature 29"
  Demux1/19 -> [HVBatCell_Temperature019]
  Demux1/3 -> [HVBatCell_Temperature003]
  [HVBatCell_Temperature053] -> BusCreator_Temp/53  "Cell Temperature 53"
  Demux1/105 -> [HVBatCell_Temperature105]
  Demux1/96 -> [HVBatCell_Temperature096]
  [HVBatCell_Temperature080] -> BusCreator_Temp/80  "Cell Temperature 80"
  [HVBatCell_Temperature069] -> BusCreator_Temp/69  "Cell Temperature 69"
  Demux1/94 -> [HVBatCell_Temperature094]
  Demux1/58 -> [HVBatCell_Temperature058]
  [HVBatCell_Temperature059] -> BusCreator_Temp/59  "Cell Temperature 59"
  [HVBatCell_Temperature068] -> BusCreator_Temp/68  "Cell Temperature 68"
  Demux1/93 -> [HVBatCell_Temperature093]
  [HVBatCell_Temperature002] -> BusCreator_Temp/2  "Cell Temperature 2"
  [HVBatCell_Temperature067] -> BusCreator_Temp/67  "Cell Temperature 67"
  [HVBatCell_Temperature019] -> BusCreator_Temp/19  "Cell Temperature 19"
  [HVBatCell_Temperature091] -> BusCreator_Temp/91  "Cell Temperature 91"
  Demux1/78 -> [HVBatCell_Temperature078]
  [HVBatCell_Temperature014] -> BusCreator_Temp/14  "Cell Temperature 14"
  [HVBatCell_Temperature030] -> BusCreator_Temp/30  "Cell Temperature 30"
  [HVBatCell_Temperature060] -> BusCreator_Temp/60  "Cell Temperature 60"
  Demux1/65 -> [HVBatCell_Temperature065]
  Demux1/52 -> [HVBatCell_Temperature052]
  [HVBatCell_Temperature079] -> BusCreator_Temp/79  "Cell Temperature 79"
  Demux1/27 -> [HVBatCell_Temperature027]
  Demux1/82 -> [HVBatCell_Temperature082]
  [HVBatCell_Temperature089] -> BusCreator_Temp/89  "Cell Temperature 89"
  Demux1/43 -> [HVBatCell_Temperature043]
  Demux1/15 -> [HVBatCell_Temperature015]
  Subtract1/1 -> temperatureCell/1
  [HVBatCell_Temperature105] -> BusCreator_Temp/106  "Cell Temperature 106"
  [HVBatCell_Temperature105] -> BusCreator_Temp/107  "Cell Temperature 107"
  [HVBatCell_Temperature105] -> BusCreator_Temp/108  "Cell Temperature 108"
  [HVBatCell_Temperature105] -> BusCreator_Temp/109  "Cell Temperature 109"
  [HVBatCell_Temperature105] -> BusCreator_Temp/110  "Cell Temperature 110"
  [HVBatCell_Temperature105] -> BusCreator_Temp/111  "Cell Temperature 111"
  [HVBatCell_Temperature105] -> BusCreator_Temp/112  "Cell Temperature 112"
  [HVBatCell_Temperature105] -> BusCreator_Temp/113  "Cell Temperature 113"
  [HVBatCell_Temperature105] -> BusCreator_Temp/114  "Cell Temperature 114"
  [HVBatCell_Temperature105] -> BusCreator_Temp/115  "Cell Temperature 115"
  [HVBatCell_Temperature105] -> BusCreator_Temp/116  "Cell Temperature 116"
  [HVBatCell_Temperature105] -> BusCreator_Temp/117  "Cell Temperature 117"
  [HVBatCell_Temperature105] -> BusCreator_Temp/118  "Cell Temperature 118"
  [HVBatCell_Temperature105] -> BusCreator_Temp/119  "Cell Temperature 119"
  [HVBatCell_Temperature105] -> BusCreator_Temp/120  "Cell Temperature 120"
  [HVBatCell_Temperature105] -> BusCreator_Temp/121  "Cell Temperature 121"
  [HVBatCell_Temperature105] -> BusCreator_Temp/122  "Cell Temperature 122"
  [HVBatCell_Temperature105] -> BusCreator_Temp/123  "Cell Temperature 123"
  [HVBatCell_Temperature105] -> BusCreator_Temp/124  "Cell Temperature 124"
  [HVBatCell_Temperature105] -> BusCreator_Temp/125  "Cell Temperature 125"
  [HVBatCell_Temperature105] -> BusCreator_Temp/126  "Cell Temperature 126"
  [HVBatCell_Temperature105] -> BusCreator_Temp/127  "Cell Temperature 127"
  [HVBatCell_Temperature105] -> BusCreator_Temp/128  "Cell Temperature 128"
  [HVBatCell_Temperature105] -> BusCreator_Temp/129  "Cell Temperature 129"
  [HVBatCell_Temperature105] -> BusCreator_Temp/130  "Cell Temperature 130"
  [HVBatCell_Temperature105] -> BusCreator_Temp/131  "Cell Temperature 131"
  [HVBatCell_Temperature105] -> BusCreator_Temp/132  "Cell Temperature 132"
  [HVBatCell_Temperature105] -> BusCreator_Temp/133  "Cell Temperature 133"
  [HVBatCell_Temperature105] -> BusCreator_Temp/134  "Cell Temperature 134"
  [HVBatCell_Temperature105] -> BusCreator_Temp/135  "Cell Temperature 135"
  [HVBatCell_Temperature105] -> BusCreator_Temp/136  "Cell Temperature 136"
  [HVBatCell_Temperature105] -> BusCreator_Temp/137  "Cell Temperature 137"
  [HVBatCell_Temperature105] -> BusCreator_Temp/138  "Cell Temperature 138"
  [HVBatCell_Temperature105] -> BusCreator_Temp/139  "Cell Temperature 139"
  [HVBatCell_Temperature105] -> BusCreator_Temp/140  "Cell Temperature 140"
  " 1"/1 -> HVBatPack_DCFCVoltage/1
  K4/1 -> Unit Delay/1
  Unit Delay/1 -> Simulink-PS Converter3/1
  " "/1 -> HVBatPack_EDMVoltage/1
  K1/1 -> Delay/1
  Delay/1 -> Simulink-PS Converter1/1
  BalCmd/1 -> Pack1/1(balancing)
  Pack1/3(socCell) -> socCell/1
  MaxChargeCurrentAllowed/1 -> Simulink-PS Converter4/1
  1/C/1 -> Subtract/2
  Discrete-Time Integrator/1 -> HVBatPrecharge_Temp/1, T(t) - T_ambient/1
  1/(R_th*C)/1 -> Subtract/1
  1/R/1 -> 1/C/1
  [HVBatPrecharge_Voltage] -> V^2/1
  V^2/1 -> 1/R/1
  Subtract/1 -> Discrete-Time Integrator/1
  T(t) - T_ambient/1 -> 1/(R_th*C)/1
  T_ambient/1 -> T(t) - T_ambient/2
  connections:
  K1 - Main Contactor/LConn3 -- Resistor1/LConn1
  K2 - Precharge Relay (-)/RConn2 -- K1 - Main Contactor/RConn4 -- Capacitor Bank/RConn1 -- Resistor2/RConn1 -- Voltage Sensor/RConn2 -- K4 Resistor (Pole 2)/RConn1
  Precharge Fuse/LConn1 -- Resistor/RConn1 -- Current Sensor/RConn2
  K3 - Precharge Relay (+)/RConn2 -- K1 - Main Contactor/RConn2 -- K4 Resistor (Pole 1)/RConn1 -- F2 - PDC Fuse/RConn1 -- F3 - EDM Fuse/RConn1
  Precharge Resistor/LConn1 -- Voltage Sensor3/LConn1 -- K3 - Precharge Relay (+)/LConn2
  Simulink-PS Converter2/RConn1 -- K2 - Precharge Relay (-)/LConn1
  Precharge Resistor/RConn1 -- Voltage Sensor3/RConn2 -- Precharge Fuse/RConn1
  Simulink-PS Converter1/RConn1 -- K1 - Main Contactor/LConn1
  Current Sensor/LConn1 -- Pack1/LConn1 -- Voltage Sensor1/LConn1
  Simulink-PS Converter/RConn1 -- K3 - Precharge Relay (+)/LConn1
  Voltage Sensor/RConn1 -- " "/LConn1
  K4 - DCFC Contactor/LConn1 -- Simulink-PS Converter3/RConn1
  "   "/LConn1 -- Current Sensor/RConn1
  Voltage Sensor1/RConn1 -- PS-Simulink 1/LConn1
  K1 - Main Contactor/LConn2 -- Resistor/LConn1
  Resistor1/RConn1 -- K2 - Precharge Relay (-)/LConn2 -- Pack1/RConn1 -- Voltage Sensor1/RConn2 -- Solver Configuration/RConn1 -- Ground/LConn1
  K4 - DCFC Contactor/LConn2 -- K4 Resistor (Pole 1)/LConn1
  K4 Resistor (Pole 2)/LConn1 -- K4 - DCFC Contactor/LConn3
  Voltage Sensor2/LConn1 -- Resistor3/LConn1 -- Controlled Current Source/LConn1 -- Capacitor/LConn1 -- K4 - DCFC Contactor/RConn2
  Voltage Sensor2/RConn2 -- Resistor3/RConn1 -- Controlled Current Source/RConn2 -- Capacitor/RConn1 -- K4 - DCFC Contactor/RConn4
  Voltage Sensor2/RConn1 -- " 1"/LConn1
  PS-Simulink Converter/LConn1 -- Voltage Sensor3/RConn1
  F3 - EDM Fuse/LConn1 -- Resistor2/LConn1 -- Voltage Sensor/LConn1 -- Capacitor Bank/LConn1
  Simulink-PS Converter4/RConn1 -- Controlled Current Source/RConn1

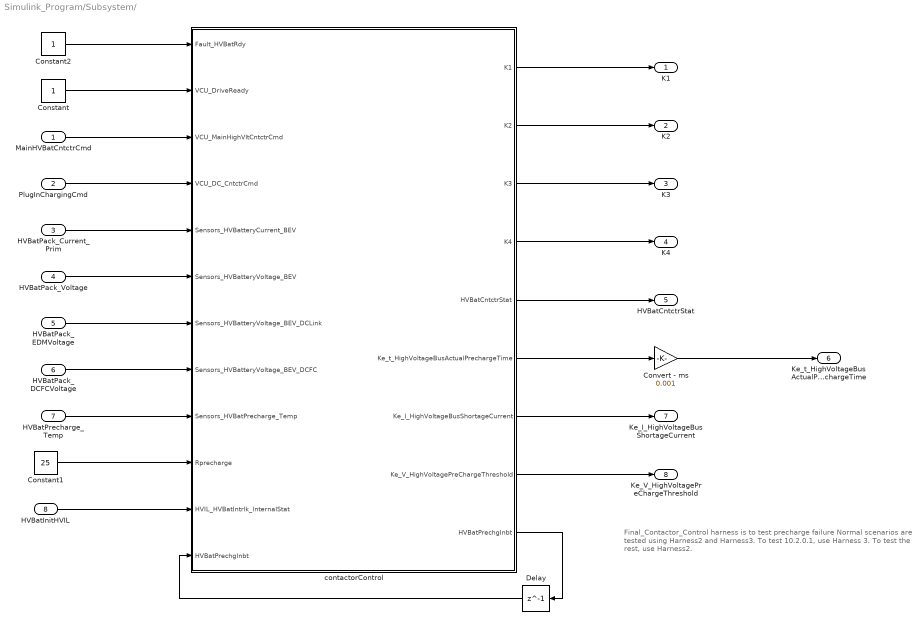
== system Simulink_Program/Subsystem/   ==
  note: Final_Contactor_Control harness is to test precharge failure
      |
      | Normal scenarios are tested using Harness2 and Harness3.
      |
      | To test 10.2.0.1, use Harness 3.
      |
      | To test the rest, use Harness2.
  MainHVBatCntctrCmd : Inport  SampleTime=0.001
  PlugInChargingCmd : Inport  Port=2  SampleTime=0.001
  HVBatPack_Current_Prim : Inport  Port=3  SampleTime=0.001
  HVBatPack_Voltage : Inport  Port=4  SampleTime=0.001
  HVBatPack_EDMVoltage : Inport  Port=5  SampleTime=0.001
  HVBatPack_DCFCVoltage : Inport  Port=6  SampleTime=0.001
  HVBatPrecharge_Temp : Inport  Port=7
  HVBatInitHVIL : Inport  Port=8
  Constant : Constant
  Constant1 : Constant  Value=25
  Constant2 : Constant
  Convert - ms : Gain  Gain=0.001
  Delay : Delay  DelayLength=1
  contactorControl : MATLAB Function  TreatAsAtomicUnit=on  SystemSampleTime=0.001
  K1 : Outport
  K2 : Outport  Port=2
  K3 : Outport  Port=3
  K4 : Outport  Port=4
  HVBatCntctrStat : Outport  Port=5
  Ke_t_HighVoltageBusActualPrechargeTime : Outport  Port=6
  Ke_I_HighVoltageBusShortageCurrent : Outport  Port=7
  Ke_V_HighVoltagePreChargeThreshold : Outport  Port=8
  wires:
  Constant1/1 -> contactorControl/10(Rprecharge)
  PlugInChargingCmd/1 -> contactorControl/4(VCU_DC_CntctrCmd)
  contactorControl/7(Ke_I_HighVoltageBusShortageCurrent) -> Ke_I_HighVoltageBusShortageCurrent/1
  MainHVBatCntctrCmd/1 -> contactorControl/3(VCU_MainHighVltCntctrCmd)
  HVBatPack_DCFCVoltage/1 -> contactorControl/8(Sensors_HVBatteryVoltage_BEV_DCFC)
  HVBatPack_EDMVoltage/1 -> contactorControl/7(Sensors_HVBatteryVoltage_BEV_DCLink)
  contactorControl/1(K1) -> K1/1
  HVBatPack_Voltage/1 -> contactorControl/6(Sensors_HVBatteryVoltage_BEV)
  HVBatInitHVIL/1 -> contactorControl/11(HVIL_HVBatIntrlk_InternalStat)
  HVBatPack_Current_Prim/1 -> contactorControl/5(Sensors_HVBatteryCurrent_BEV)
  HVBatPrecharge_Temp/1 -> contactorControl/9(Sensors_HVBatPrecharge_Temp)
  contactorControl/8(Ke_V_HighVoltagePreChargeThreshold) -> Ke_V_HighVoltagePreChargeThreshold/1
  Delay/1 -> contactorControl/12("HVBatPrechgInbt ")
  contactorControl/2(K2) -> K2/1
  contactorControl/3(K3) -> K3/1
  contactorControl/4(K4) -> K4/1
  contactorControl/5(HVBatCntctrStat) -> HVBatCntctrStat/1
  contactorControl/6(Ke_t_HighVoltageBusActualPrechargeTime) -> Convert - ms/1
  contactorControl/9(HVBatPrechgInbt) -> Delay/1
  Convert - ms/1 -> Ke_t_HighVoltageBusActualPrechargeTime/1
  Constant2/1 -> contactorControl/1(Fault_HVBatRdy)
  Constant/1 -> contactorControl/2(VCU_DriveReady)
